simulink model "BMSModel"  (R2024b)
settings: Solver=VariableStepAuto  StartTime=0.0  StopTime=+inf  RelTol=1e-3
values from neighbouring files (symbols this model uses):
  CellCurrentLimitThreshold_Fault = 50    [startInit.m]
  CellCurrentLimitThreshold_Warning = 20    [startInit.m]
  CellTemperatureLimitThreshold_Fault = 65    [startInit.m]
  CellTemperatureLimitThreshold_Warning = 50    [startInit.m]
  CellVoltageLimitHigh_Fault = 4.25    [startInit.m]
  CellVoltageLimitHigh_Warning = 4.21    [startInit.m]
  CellVoltageLimitLow_Fault = 2.65    [startInit.m]
  CellVoltageLimitLow_Warning = 2.70    (= 2.7)    [startInit.m]
  ChargerParRes = 10000    [startInit.m]
  ChargerRes = 0    [startInit.m]
  DisChargerRes = 250    [startInit.m]
  DisInverterRes = 250    [startInit.m]
  DrivetrainEnDelay = 0.1    [startInit.m]
  InverterParRes = 10000    [startInit.m]
  InverterRes = 0    [startInit.m]
  PreChargerRes = 1000    [startInit.m]
  PreInverterRes = 1000    [startInit.m]
  VbattMin = 1    [startInit.m]
  VbattThersholdChrg = 0.8    [startInit.m]
  VbattThresholdDis = 0.2    [startInit.m]
undefined symbols (not defined in the file or in its folder): ContactorCmd, DisChrgCmd, DrivetrainEn, PassiveBalancingRes, PreChrgCmd, sigbuilder_block
libraries: fl_lib x135, nesl_utility x91, deeplib x11, batteryecm_lib x10, ee_lib x10
(part 2 of 13)

== system BMSModel/Electric Vehicul Model/HV Battery/Battery Pack/Battery Model ==
  AmbTemp : Inport
  ShortFaultCmd : Inport  Port=2
  OpenFaultCmd : Inport  Port=3
  PassiveControlBalCmd : Inport  Port=4
  Battery_Model : Subsystem
  Resistor : fl_lib/Electrical/Electrical Elements/Resistor  R=0.05 Ohm  i_specify=off  i_priority=None  i=0 A  i_nominal_specify=off  i_nominal_value=1  i_nominal_unit=A  v_specify=off  v_priority=None  v=0 V  v_nominal_specify=off  v_nominal_value=1  v_nominal_unit=V
  Resistor1 : fl_lib/Electrical/Electrical Elements/Resistor  R=0.05 Ohm  i_specify=off  i_priority=None  i=0 A  i_nominal_specify=off  i_nominal_value=1  i_nominal_unit=A  v_specify=off  v_priority=None  v=0 V  v_nominal_specify=off  v_nominal_value=1  v_nominal_unit=V
  Subsystem1 : Subsystem
  BattMod+ : PMIOPort  Side=Right
  BattMod- : PMIOPort  Port=2  Side=Right
  TCellVector : Outport
  RealSOCVector : Outport  Port=2
  VoltageVector : Outport  Port=3
  CurrentCellVector : Outport  Port=4
  wires:
  OpenFaultCmd/1 -> Battery_Model/3(OpenFaultCmd)
  Battery_Model/1(TCellVector) -> TCellVector/1
  Subsystem1/1(VoltageVector) -> VoltageVector/1
  Battery_Model/2(RealSocVector) -> RealSOCVector/1
  Battery_Model/3(CurrentCellVector) -> CurrentCellVector/1
  AmbTemp/1 -> Battery_Model/1(AmbTemp)
  PassiveControlBalCmd/1 -> Subsystem1/1(PassiveControlBalCmd1)
  ShortFaultCmd/1 -> Battery_Model/2(ShortFaultCmd)
  connections:
  Subsystem1/LConn7 -- Battery_Model/RConn7
  Subsystem1/LConn1 -- Resistor/LConn1 -- Battery_Model/RConn1
  Subsystem1/LConn11 -- Resistor1/LConn1 -- Battery_Model/RConn11
  Subsystem1/LConn9 -- Battery_Model/RConn9
  Subsystem1/LConn6 -- Battery_Model/RConn6
  Subsystem1/LConn10 -- Battery_Model/RConn10
  Subsystem1/LConn4 -- Battery_Model/RConn4
  Subsystem1/LConn5 -- Battery_Model/RConn5
  Subsystem1/LConn8 -- Battery_Model/RConn8
  Subsystem1/LConn3 -- Battery_Model/RConn3
  Subsystem1/LConn2 -- Battery_Model/RConn2
  BattMod+/RConn1 -- Resistor/RConn1
  BattMod-/RConn1 -- Resistor1/RConn1

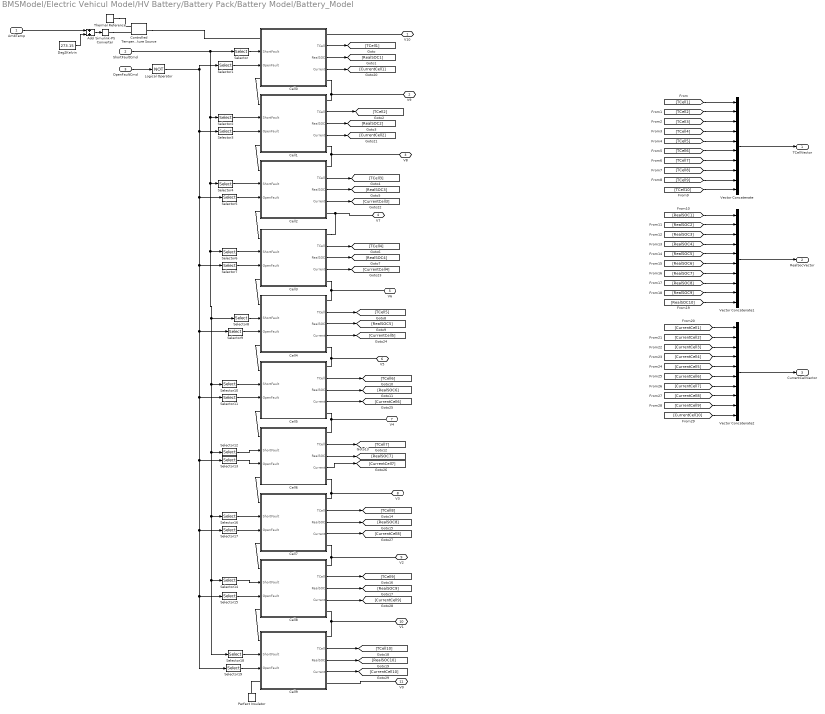
== system BMSModel/Electric Vehicul Model/HV Battery/Battery Pack/Battery Model/Battery_Model ==
  AmbTemp : Inport
  ShortFaultCmd : Inport  Port=2
  OpenFaultCmd : Inport  Port=3  OutDataTypeStr=boolean
  Add : Sum
  Cell0 : Subsystem
      settings: PortSchema="{\"entries\":[{\"content\":{\"sides\":[{\"content\":{\"connectorIds\":[\"LConn1\",\"In1\",\"In2\",\"LConn2\"]},\"type\":\"ConnectorPlacement.EquallySpacedRectSide\",\"uuid\":\"beb41537-88d3-447c-be57-cb35454cee64\"},{\"content\":{\"connectorIds\":[\"RConn1\",\"Out1\",\"Out2\",\"Out3\",\"RConn2\"],\"side\":\"RIGHT\"},\"type\":\"ConnectorPlacement.EquallySpacedRectSide\",\"uuid\":\"6a20e019-ad86-49c6-8fd8-d46a955d3cd5\"},{\"content\":{\"side\":\"TOP\"},\"type\":\"ConnectorPlacement.EquallySpacedRectSide\",\"uuid\":\"023fac5e-8c8d-4c82-a029-53fce6daf92f\"}]},\"type\":\"ConnectorPlacement.EquallySpacedConnector\",\"uuid\":\"8f1c4e8b-ec41-4c09-b75c-bd6301f7044f\"}],\"packageUris\":[\"http://schema.mathworks.com/mf0/Connector/R2020b\"],\"version\":\"1.0\"}"
      mask Display: image('.\icons\images.jpg');
  Cell1 : Subsystem
      settings: PortSchema="{\"entries\":[{\"content\":{\"sides\":[{\"content\":{\"connectorIds\":[\"LConn1\",\"In1\",\"In2\",\"LConn2\"]},\"type\":\"ConnectorPlacement.EquallySpacedRectSide\",\"uuid\":\"beb41537-88d3-447c-be57-cb35454cee64\"},{\"content\":{\"connectorIds\":[\"RConn1\",\"Out1\",\"Out2\",\"Out3\",\"RConn2\"],\"side\":\"RIGHT\"},\"type\":\"ConnectorPlacement.EquallySpacedRectSide\",\"uuid\":\"6a20e019-ad86-49c6-8fd8-d46a955d3cd5\"},{\"content\":{\"side\":\"TOP\"},\"type\":\"ConnectorPlacement.EquallySpacedRectSide\",\"uuid\":\"023fac5e-8c8d-4c82-a029-53fce6daf92f\"}]},\"type\":\"ConnectorPlacement.EquallySpacedConnector\",\"uuid\":\"8f1c4e8b-ec41-4c09-b75c-bd6301f7044f\"}],\"packageUris\":[\"http://schema.mathworks.com/mf0/Connector/R2020b\"],\"version\":\"1.0\"}"
      mask Display: image('.\icons\images.jpg');
  Cell2 : Subsystem
      settings: PortSchema="{\"entries\":[{\"content\":{\"sides\":[{\"content\":{\"connectorIds\":[\"LConn1\",\"In1\",\"In2\",\"LConn2\"]},\"type\":\"ConnectorPlacement.EquallySpacedRectSide\",\"uuid\":\"beb41537-88d3-447c-be57-cb35454cee64\"},{\"content\":{\"connectorIds\":[\"RConn1\",\"Out1\",\"Out2\",\"Out3\",\"RConn2\"],\"side\":\"RIGHT\"},\"type\":\"ConnectorPlacement.EquallySpacedRectSide\",\"uuid\":\"6a20e019-ad86-49c6-8fd8-d46a955d3cd5\"},{\"content\":{\"side\":\"TOP\"},\"type\":\"ConnectorPlacement.EquallySpacedRectSide\",\"uuid\":\"023fac5e-8c8d-4c82-a029-53fce6daf92f\"}]},\"type\":\"ConnectorPlacement.EquallySpacedConnector\",\"uuid\":\"8f1c4e8b-ec41-4c09-b75c-bd6301f7044f\"}],\"packageUris\":[\"http://schema.mathworks.com/mf0/Connector/R2020b\"],\"version\":\"1.0\"}"
      mask Display: image('D:\BMS-AI\Dev_BMS_with_AI\icons\images.jpg');
  Cell3 : Subsystem
      settings: PortSchema="{\"entries\":[{\"content\":{\"sides\":[{\"content\":{\"connectorIds\":[\"LConn1\",\"In1\",\"In2\",\"LConn2\"]},\"type\":\"ConnectorPlacement.EquallySpacedRectSide\",\"uuid\":\"beb41537-88d3-447c-be57-cb35454cee64\"},{\"content\":{\"connectorIds\":[\"RConn1\",\"Out1\",\"Out2\",\"Out3\",\"RConn2\"],\"side\":\"RIGHT\"},\"type\":\"ConnectorPlacement.EquallySpacedRectSide\",\"uuid\":\"6a20e019-ad86-49c6-8fd8-d46a955d3cd5\"},{\"content\":{\"side\":\"TOP\"},\"type\":\"ConnectorPlacement.EquallySpacedRectSide\",\"uuid\":\"023fac5e-8c8d-4c82-a029-53fce6daf92f\"}]},\"type\":\"ConnectorPlacement.EquallySpacedConnector\",\"uuid\":\"8f1c4e8b-ec41-4c09-b75c-bd6301f7044f\"}],\"packageUris\":[\"http://schema.mathworks.com/mf0/Connector/R2020b\"],\"version\":\"1.0\"}"
  Cell4 : Subsystem
      settings: PortSchema="{\"entries\":[{\"content\":{\"sides\":[{\"content\":{\"connectorIds\":[\"LConn1\",\"In1\",\"In2\",\"LConn2\"]},\"type\":\"ConnectorPlacement.EquallySpacedRectSide\",\"uuid\":\"beb41537-88d3-447c-be57-cb35454cee64\"},{\"content\":{\"connectorIds\":[\"RConn1\",\"Out1\",\"Out2\",\"Out3\",\"RConn2\"],\"side\":\"RIGHT\"},\"type\":\"ConnectorPlacement.EquallySpacedRectSide\",\"uuid\":\"6a20e019-ad86-49c6-8fd8-d46a955d3cd5\"},{\"content\":{\"side\":\"TOP\"},\"type\":\"ConnectorPlacement.EquallySpacedRectSide\",\"uuid\":\"023fac5e-8c8d-4c82-a029-53fce6daf92f\"}]},\"type\":\"ConnectorPlacement.EquallySpacedConnector\",\"uuid\":\"8f1c4e8b-ec41-4c09-b75c-bd6301f7044f\"}],\"packageUris\":[\"http://schema.mathworks.com/mf0/Connector/R2020b\"],\"version\":\"1.0\"}"
  Cell5 : Subsystem
      settings: PortSchema="{\"entries\":[{\"content\":{\"sides\":[{\"content\":{\"connectorIds\":[\"LConn1\",\"In1\",\"In2\",\"LConn2\"]},\"type\":\"ConnectorPlacement.EquallySpacedRectSide\",\"uuid\":\"beb41537-88d3-447c-be57-cb35454cee64\"},{\"content\":{\"connectorIds\":[\"RConn1\",\"Out1\",\"Out2\",\"Out3\",\"RConn2\"],\"side\":\"RIGHT\"},\"type\":\"ConnectorPlacement.EquallySpacedRectSide\",\"uuid\":\"6a20e019-ad86-49c6-8fd8-d46a955d3cd5\"},{\"content\":{\"side\":\"TOP\"},\"type\":\"ConnectorPlacement.EquallySpacedRectSide\",\"uuid\":\"023fac5e-8c8d-4c82-a029-53fce6daf92f\"}]},\"type\":\"ConnectorPlacement.EquallySpacedConnector\",\"uuid\":\"8f1c4e8b-ec41-4c09-b75c-bd6301f7044f\"}],\"packageUris\":[\"http://schema.mathworks.com/mf0/Connector/R2020b\"],\"version\":\"1.0\"}"
  Cell6 : Subsystem
      settings: PortSchema="{\"entries\":[{\"content\":{\"sides\":[{\"content\":{\"connectorIds\":[\"LConn1\",\"In1\",\"In2\",\"LConn2\"]},\"type\":\"ConnectorPlacement.EquallySpacedRectSide\",\"uuid\":\"beb41537-88d3-447c-be57-cb35454cee64\"},{\"content\":{\"connectorIds\":[\"RConn1\",\"Out1\",\"Out2\",\"Out3\",\"RConn2\"],\"side\":\"RIGHT\"},\"type\":\"ConnectorPlacement.EquallySpacedRectSide\",\"uuid\":\"6a20e019-ad86-49c6-8fd8-d46a955d3cd5\"},{\"content\":{\"side\":\"TOP\"},\"type\":\"ConnectorPlacement.EquallySpacedRectSide\",\"uuid\":\"023fac5e-8c8d-4c82-a029-53fce6daf92f\"}]},\"type\":\"ConnectorPlacement.EquallySpacedConnector\",\"uuid\":\"8f1c4e8b-ec41-4c09-b75c-bd6301f7044f\"}],\"packageUris\":[\"http://schema.mathworks.com/mf0/Connector/R2020b\"],\"version\":\"1.0\"}"
  Cell7 : Subsystem
      settings: PortSchema="{\"entries\":[{\"content\":{\"sides\":[{\"content\":{\"connectorIds\":[\"LConn1\",\"In1\",\"In2\",\"LConn2\"]},\"type\":\"ConnectorPlacement.EquallySpacedRectSide\",\"uuid\":\"beb41537-88d3-447c-be57-cb35454cee64\"},{\"content\":{\"connectorIds\":[\"RConn1\",\"Out1\",\"Out2\",\"Out3\",\"RConn2\"],\"side\":\"RIGHT\"},\"type\":\"ConnectorPlacement.EquallySpacedRectSide\",\"uuid\":\"6a20e019-ad86-49c6-8fd8-d46a955d3cd5\"},{\"content\":{\"side\":\"TOP\"},\"type\":\"ConnectorPlacement.EquallySpacedRectSide\",\"uuid\":\"023fac5e-8c8d-4c82-a029-53fce6daf92f\"}]},\"type\":\"ConnectorPlacement.EquallySpacedConnector\",\"uuid\":\"8f1c4e8b-ec41-4c09-b75c-bd6301f7044f\"}],\"packageUris\":[\"http://schema.mathworks.com/mf0/Connector/R2020b\"],\"version\":\"1.0\"}"
  Cell8 : Subsystem
      settings: PortSchema="{\"entries\":[{\"content\":{\"sides\":[{\"content\":{\"connectorIds\":[\"LConn1\",\"In1\",\"In2\",\"LConn2\"]},\"type\":\"ConnectorPlacement.EquallySpacedRectSide\",\"uuid\":\"beb41537-88d3-447c-be57-cb35454cee64\"},{\"content\":{\"connectorIds\":[\"RConn1\",\"Out1\",\"Out2\",\"Out3\",\"RConn2\"],\"side\":\"RIGHT\"},\"type\":\"ConnectorPlacement.EquallySpacedRectSide\",\"uuid\":\"6a20e019-ad86-49c6-8fd8-d46a955d3cd5\"},{\"content\":{\"side\":\"TOP\"},\"type\":\"ConnectorPlacement.EquallySpacedRectSide\",\"uuid\":\"023fac5e-8c8d-4c82-a029-53fce6daf92f\"}]},\"type\":\"ConnectorPlacement.EquallySpacedConnector\",\"uuid\":\"8f1c4e8b-ec41-4c09-b75c-bd6301f7044f\"}],\"packageUris\":[\"http://schema.mathworks.com/mf0/Connector/R2020b\"],\"version\":\"1.0\"}"
  Cell9 : Subsystem
      settings: PortSchema="{\"entries\":[{\"content\":{\"sides\":[{\"content\":{\"connectorIds\":[\"LConn1\",\"In1\",\"In2\",\"LConn2\"]},\"type\":\"ConnectorPlacement.EquallySpacedRectSide\",\"uuid\":\"beb41537-88d3-447c-be57-cb35454cee64\"},{\"content\":{\"connectorIds\":[\"RConn1\",\"Out1\",\"Out2\",\"Out3\",\"RConn2\"],\"side\":\"RIGHT\"},\"type\":\"ConnectorPlacement.EquallySpacedRectSide\",\"uuid\":\"6a20e019-ad86-49c6-8fd8-d46a955d3cd5\"},{\"content\":{\"side\":\"TOP\"},\"type\":\"ConnectorPlacement.EquallySpacedRectSide\",\"uuid\":\"023fac5e-8c8d-4c82-a029-53fce6daf92f\"}]},\"type\":\"ConnectorPlacement.EquallySpacedConnector\",\"uuid\":\"8f1c4e8b-ec41-4c09-b75c-bd6301f7044f\"}],\"packageUris\":[\"http://schema.mathworks.com/mf0/Connector/R2020b\"],\"version\":\"1.0\"}"
  Controlled Temperature Source : fl_lib/Thermal/Thermal Sources/Controlled Temperature Source
  Deg2Kelvin : Constant  Value=273.15
  Logical Operator : Logic  Operator=NOT  AllPortsSameDT=off  OutDataTypeStr=boolean
  Perfect Insulator : fl_lib/Thermal/Thermal Elements/Perfect Insulator  T_specify=off  T_priority=None  T=293.15 K  T_nominal_specify=off  T_nominal_value=1  T_nominal_unit=K
  Selector : Selector  InputPortWidth=10  IndexOptions=Index vector (dialog)  Indices=1  OutputSizes=1
  Selector1 : Selector  InputPortWidth=10  IndexOptions=Index vector (dialog)  Indices=1  OutputSizes=1
  Selector10 : Selector  InputPortWidth=10  IndexOptions=Index vector (dialog)  Indices=6  OutputSizes=1
  Selector11 : Selector  InputPortWidth=10  IndexOptions=Index vector (dialog)  Indices=6  OutputSizes=1
  Selector12 : Selector  InputPortWidth=10  IndexOptions=Index vector (dialog)  Indices=7  OutputSizes=1
  Selector13 : Selector  InputPortWidth=10  IndexOptions=Index vector (dialog)  Indices=7  OutputSizes=1
  Selector14 : Selector  InputPortWidth=10  IndexOptions=Index vector (dialog)  Indices=9  OutputSizes=1
  Selector15 : Selector  InputPortWidth=10  IndexOptions=Index vector (dialog)  Indices=9  OutputSizes=1
  Selector16 : Selector  InputPortWidth=10  IndexOptions=Index vector (dialog)  Indices=8  OutputSizes=1
  Selector17 : Selector  InputPortWidth=10  IndexOptions=Index vector (dialog)  Indices=8  OutputSizes=1
  Selector18 : Selector  InputPortWidth=10  IndexOptions=Index vector (dialog)  Indices=10  OutputSizes=1
  Selector19 : Selector  InputPortWidth=10  IndexOptions=Index vector (dialog)  Indices=10  OutputSizes=1
  Selector2 : Selector  InputPortWidth=10  IndexOptions=Index vector (dialog)  Indices=2  OutputSizes=1
  Selector3 : Selector  InputPortWidth=10  IndexOptions=Index vector (dialog)  Indices=2  OutputSizes=1
  Selector4 : Selector  InputPortWidth=10  IndexOptions=Index vector (dialog)  Indices=3  OutputSizes=1
  Selector5 : Selector  InputPortWidth=10  IndexOptions=Index vector (dialog)  Indices=3  OutputSizes=1
  Selector6 : Selector  InputPortWidth=10  IndexOptions=Index vector (dialog)  Indices=4  OutputSizes=1
  Selector7 : Selector  InputPortWidth=10  IndexOptions=Index vector (dialog)  Indices=4  OutputSizes=1
  Selector8 : Selector  InputPortWidth=10  IndexOptions=Index vector (dialog)  Indices=5  OutputSizes=1
  Selector9 : Selector  InputPortWidth=10  IndexOptions=Index vector (dialog)  Indices=5  OutputSizes=1
  Simulink-PS Converter : nesl_utility/Simulink-PS Converter  PhysicalDomain=network_engine_domain  SubClassName=ps_input  LeftPortType=input  RightPortType=output  PseudoPeriodic=off  Frequencies=[]  NoiseDistribution=none  NoiseParameters=[]  Unit=1  AffineConversion=off  FilteringAndDerivatives=provide  SimscapeFilterOrder=1  InputFilterTimeConstant=0.001  UdotUserProvided=0
  Thermal Reference : fl_lib/Thermal/Thermal Elements/Thermal Reference
  Vector Concatenate : Concatenate  NumInputs=10
  Vector Concatenate1 : Concatenate  NumInputs=10
  Vector Concatenate2 : Concatenate  NumInputs=10
  V10 : PMIOPort  Side=Right
  V9 : PMIOPort  Port=2  Side=Right
  V8 : PMIOPort  Port=3  Side=Right
  V7 : PMIOPort  Port=4  Side=Right
  V6 : PMIOPort  Port=5  Side=Right
  V5 : PMIOPort  Port=6  Side=Right
  V4 : PMIOPort  Port=7  Side=Right
  V3 : PMIOPort  Port=8  Side=Right
  V2 : PMIOPort  Port=9  Side=Right
  V1 : PMIOPort  Port=10  Side=Right
  V0 : PMIOPort  Port=11  Side=Right
  TCellVector : Outport
  RealSocVector : Outport  Port=2
  CurrentCellVector : Outport  Port=3
  wires:
  Deg2Kelvin/1 -> Add/2
  Add/1 -> Simulink-PS Converter/1
  AmbTemp/1 -> Add/1
  Cell0/1(TCell) -> [TCell1]
  Cell0/2(RealSOC) -> [RealSOC1]
  Cell0/3(Current) -> [CurrentCell1]
  Cell1/1(TCell) -> [TCell2]
  Cell1/2(RealSOC) -> [RealSOC2]
  Cell1/3(Current) -> [CurrentCell2]
  Cell2/1(TCell) -> [TCell3]
  Cell2/2(RealSOC) -> [RealSOC3]
  Cell2/3(Current) -> [CurrentCell3]
  Cell3/1(TCell) -> [TCell4]
  Cell3/2(RealSOC) -> [RealSOC4]
  Cell3/3(Current) -> [CurrentCell4]
  Cell4/1(TCell) -> [TCell5]
  Cell4/2(RealSOC) -> [RealSOC5]
  Cell4/3(Current) -> [CurrentCell5]
  Cell5/1(TCell) -> [TCell6]
  Cell5/2(RealSOC) -> [RealSOC6]
  Cell5/3(Current) -> [CurrentCell6]
  Cell6/1(TCell) -> [TCell7]
  Cell6/2(RealSOC) -> [RealSOC7]
  Cell6/3(Current) -> [CurrentCell7]
  Cell7/2(RealSOC) -> [RealSOC8]
  Cell7/1(TCell) -> [TCell8]
  Cell7/3(Current) -> [CurrentCell8]
  Cell8/2(RealSOC) -> [RealSOC9]
  Cell8/1(TCell) -> [TCell9]
  Cell8/3(Current) -> [CurrentCell9]
  Cell9/2(RealSOC) -> [RealSOC10]
  Cell9/1(TCell) -> [TCell10]
  Cell9/3(Current) -> [CurrentCell10]
  OpenFaultCmd/1 -> Logical Operator/1
  Logical Operator/1 -> Selector3/1, Selector5/1, Selector7/1, Selector19/1, Selector15/1, Selector17/1, Selector13/1, Selector11/1, Selector9/1, Selector1/1
  ShortFaultCmd/1 -> Selector6/1, Selector8/1, Selector10/1, Selector18/1, Selector14/1, Selector16/1, Selector12/1, Selector4/1, Selector2/1, Selector/1
  Selector/1 -> Cell0/1(ShortFault)
  Selector1/1 -> Cell0/2(OpenFault)
  Selector2/1 -> Cell1/1(ShortFault)
  Selector3/1 -> Cell1/2(OpenFault)
  Selector4/1 -> Cell2/1(ShortFault)
  Selector5/1 -> Cell2/2(OpenFault)
  Selector6/1 -> Cell3/1(ShortFault)
  Selector7/1 -> Cell3/2(OpenFault)
  Selector8/1 -> Cell4/1(ShortFault)
  Selector9/1 -> Cell4/2(OpenFault)
  Selector10/1 -> Cell5/1(ShortFault)
  Selector11/1 -> Cell5/2(OpenFault)
  Selector12/1 -> Cell6/1(ShortFault)
  Selector13/1 -> Cell6/2(OpenFault)
  Selector16/1 -> Cell7/1(ShortFault)
  Selector17/1 -> Cell7/2(OpenFault)
  Selector14/1 -> Cell8/1(ShortFault)
  Selector15/1 -> Cell8/2(OpenFault)
  Selector18/1 -> Cell9/1(ShortFault)
  Selector19/1 -> Cell9/2(OpenFault)
  Vector Concatenate/1 -> TCellVector/1
  [TCell1] -> Vector Concatenate/1
  [TCell2] -> Vector Concatenate/2
  [TCell3] -> Vector Concatenate/3
  [TCell4] -> Vector Concatenate/4
  [TCell5] -> Vector Concatenate/5
  [TCell6] -> Vector Concatenate/6
  [TCell7] -> Vector Concatenate/7
  [TCell8] -> Vector Concatenate/8
  [TCell9] -> Vector Concatenate/9
  [TCell10] -> Vector Concatenate/10
  Vector Concatenate1/1 -> RealSocVector/1
  [RealSOC1] -> Vector Concatenate1/1
  [RealSOC2] -> Vector Concatenate1/2
  [RealSOC3] -> Vector Concatenate1/3
  [RealSOC4] -> Vector Concatenate1/4
  [RealSOC5] -> Vector Concatenate1/5
  [RealSOC6] -> Vector Concatenate1/6
  [RealSOC7] -> Vector Concatenate1/7
  [RealSOC8] -> Vector Concatenate1/8
  [RealSOC9] -> Vector Concatenate1/9
  [RealSOC10] -> Vector Concatenate1/10
  Vector Concatenate2/1 -> CurrentCellVector/1
  [CurrentCell1] -> Vector Concatenate2/1
  [CurrentCell2] -> Vector Concatenate2/2
  [CurrentCell3] -> Vector Concatenate2/3
  [CurrentCell4] -> Vector Concatenate2/4
  [CurrentCell5] -> Vector Concatenate2/5
  [CurrentCell6] -> Vector Concatenate2/6
  [CurrentCell7] -> Vector Concatenate2/7
  [CurrentCell8] -> Vector Concatenate2/8
  [CurrentCell9] -> Vector Concatenate2/9
  [CurrentCell10] -> Vector Concatenate2/10
  connections:
  Simulink-PS Converter/RConn1 -- Controlled Temperature Source/RConn1
  Thermal Reference/LConn1 -- Controlled Temperature Source/RConn2
  Cell0/LConn2 -- Cell1/LConn1
  Cell0/RConn2 -- V9/RConn1 -- Cell1/RConn1
  Cell1/LConn2 -- Cell2/LConn1
  Cell1/RConn2 -- Cell2/RConn1 -- V8/RConn1
  Cell4/LConn2 -- Cell5/LConn1
  Cell3/RConn2 -- V6/RConn1 -- Cell4/RConn1
  Cell4/RConn2 -- V5/RConn1 -- Cell5/RConn1
  Cell3/LConn2 -- Cell4/LConn1
  Cell6/LConn2 -- Cell7/LConn1
  Cell6/RConn2 -- V3/RConn1 -- Cell7/RConn1
  Cell7/RConn2 -- V2/RConn1 -- Cell8/RConn1
  Cell7/LConn2 -- Cell8/LConn1
  V10/RConn1 -- Cell0/RConn1
  V0/RConn1 -- Cell9/RConn2
  V7/RConn1 -- Cell3/RConn1 -- Cell2/RConn2
  Cell2/LConn2 -- Cell3/LConn1
  Cell5/RConn2 -- Cell6/RConn1 -- V4/RConn1
  Cell5/LConn2 -- Cell6/LConn1
  Cell9/RConn1 -- V1/RConn1 -- Cell8/RConn2
  Cell8/LConn2 -- Cell9/LConn1
  Controlled Temperature Source/LConn1 -- Cell0/LConn1
  Perfect Insulator/LConn1 -- Cell9/LConn2

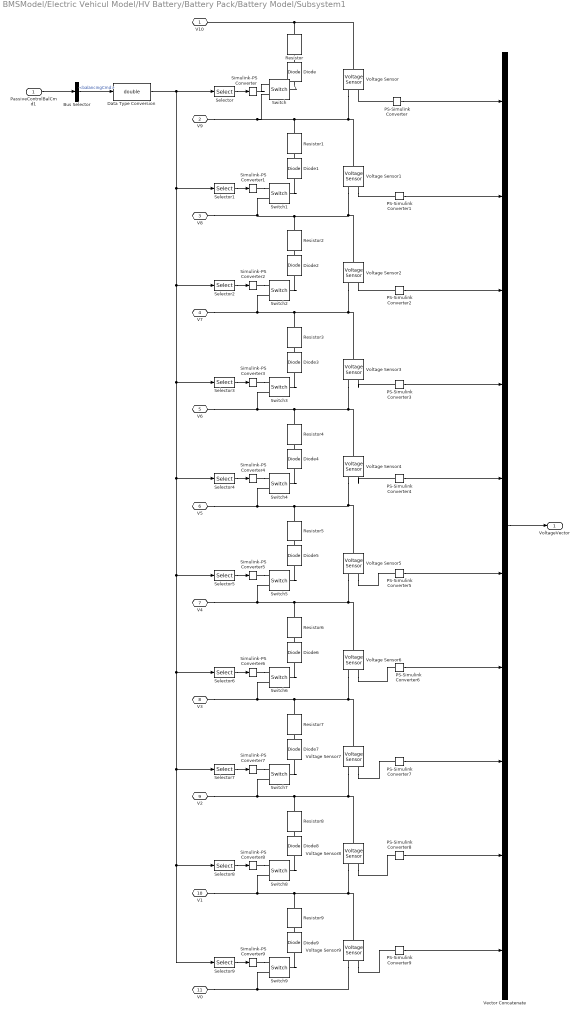
== system BMSModel/Electric Vehicul Model/HV Battery/Battery Pack/Battery Model/Subsystem1 ==
  PassiveControlBalCmd1 : Inport  OutDataTypeStr=boolean
  Bus Selector : BusSelector  OutputSignals=Balancing.balancingCmd
  Data Type Conversion : DataTypeConversion  OutDataTypeStr=double  SaturateOnIntegerOverflow=off  RndMeth=Floor
  Diode{,1..9} (10 alike) : ee_lib/Semiconductors & Converters/Diode  ModelType=ee.enum.diode.modelType.pwl  TableType=ee.enum.diode.tableType.IfMat  IfMat=[.07, .12, .19, 1.75, 4.24, 7.32, 11.2; .16, .3, .72, 2.14, 4.02, 6.35, 9.12] A  VfMat=[.9, 1.15, 1.25, 1.5, 1.75, 2.17, 2.6, 2.85; .58, .68, .75, 1.1, 1.38, 1.77, 2.27, 2.7] V  TjVec=[25, 125] degC  VfVec=[.5, .7, .9, 1.3, 1.7, 2.1, 2.5] V  IfVec=[.1, .2, .5, 1, 2, 4, 7, 10] A  IrMat=[1e-06, 2e-06, 5e-06, 1e-05, 2.5e-05, 5e-05, .0001; 2e-06, 5e-06, 1e-05, 4e-05, 8e-05, .0002, .0005] A  VrMat=[10, 100, 200, 250, 300, 325, 350; 10, 70, 160, 220, 270, 300, 320] V  VrVec=[10, 20, 40, 50, 70, 80, 100] V  IrVec=[1e-06, 2e-06, 5e-06, 1e-05, 2e-05, 5e-05, .0001] A  Vf=0.6 V  Ron=0.3 Ohm  Goff=1e-8 S  prmExp=ee.enum.diode.expParam.useTwo  I12=[.0137, .545] A  V12=[.6, .7] V  RS=0 Ohm  Tmeas=25 degC  IS=1e-12 A  ec=1  I1=0.0137 A  V1=0.6 V  N_series=1  N_parallel=1  ZenerEnable=false  Rz=0.3 Ohm  BV=inf V  C_param=ee.enum.diode.capParam.fixed  CJ=5 pF  CJ0=5 pF  Vvec=[.1, 10, 100] V  Cvec=[3.5, 1, .4] pF  VJ=1 V  Mgrad=0.5  FC=0.5  Q_param=ee.enum.diode.recoveryParam.off  iRM=-7.15 A  iF=4 A  didt=-750 A/us  trr_factor=3  trr=115 ns  Qrr=150 s*nA  Vr=-600 V  Ls=150 nH  Erec=0.03 J  TT=50 ns  tau=100 ns  T_param=ee.enum.diode.temperatureParam.off  Tdevice=25 degC  I1_T2=0.245 A  V1_T2=0.5 V  IS_T2=1.25e-7 A  Tmeas2=125 degC  XTI_param=ee.enum.diode.xtiParam.pn  XTI=3  EG_param=ee.enum.diode.egapParam.material_si  EG=1.11 eV  dBVdT=0 V/K  fault_action=simscape.enum.assert.action.none  t_fault=inf s  enable_fault_input=simscape.enum.onoff.off  i_specify=off  i_priority=None  i=0 A  i_nominal_specify=off  i_nominal_value=1  i_nominal_unit=A  v_specify=off  v_priority=None  v=0 V  v_nominal_specify=off  v_nominal_value=1  v_nominal_unit=V  v_capacitor_specify=off  v_capacitor_priority=None  v_capacitor=0 V  v_capacitor_nominal_specify=off  v_capacitor_nominal_value=1  v_capacitor_nominal_unit=V  Qj_specify=off  Qj_priority=High  Qj=0 C  Qj_nominal_specify=off  Qj_nominal_value=1  Qj_nominal_unit=C  i_diode_specify=off  i_diode_priority=None  i_diode=0 A  i_diode_nominal_specify=off  i_diode_nominal_value=1  i_diode_nominal_unit=A  v_diode_specify=off  v_diode_priority=None  v_diode=0 V  v_diode_nominal_specify=off  v_diode_nominal_value=1  v_diode_nominal_unit=V  i_capacitor_specify=off  i_capacitor_priority=None  i_capacitor=0 A  i_capacitor_nominal_specify=off  i_capacitor_nominal_value=1  i_capacitor_nominal_unit=A  power_dissipated_specify=off  power_dissipated_priority=None  power_dissipated=0 W  power_dissipated_nominal_specify=off  power_dissipated_nominal_value=1  power_dissipated_nominal_unit=W  fidelity_level=ee.enum.diode.fidelity.includeChargeDyn  rec_loss_model=ee.enum.diode.reverseRecoveryModel.fixed  ErecVal=0 J  scaleWithIandV=true  ErecMat=zeros(2, 3) J  TjrecVec=[298.15, 398.15] K  IfrecVec=[.1, 1, 10] A  IrecVal=10 A  VrecVal=10 V  tFilter=1 / 1000 / 100 s  thermal_network_parameterization=ee.enum.thermalNetworkTopology.cauer  Rth=[0, 10] K/W  Rth_Cauer=[1, 3, 10] K/W  Rth_Foster=[4, 6] K/W  thermal_mass_parameterization=ee.enum.thermalNetwork.timeconstant  thermal_mass=[0, 1] J/K  thermal_mass_Cauer=[.1, .3, 1] J/K  thermal_mass_Foster=[1.5, 3] J/K  thermal_time_constant=[0, 10] s  thermal_time_constant_Cauer=[1, 3, 10] s  thermal_time_constant_Foster=[6, 18] s  thermal_mass_initial_temperatures=[25, 25] degC  thermal_mass_initial_temperatures_Cauer=[25, 25, 25] degC  junction_thermal_mass=0.01 J/K  Tvec_initial_Foster=[25, 25, 25, 25] degC  no_thermal_network=0  junction_temperature_specify=off  junction_temperature_priority=None  junction_temperature=25 degC  junction_temperature_nominal_specify=off  junction_temperature_nominal_value=1  junction_temperature_nominal_unit=degC
  PS-Simulink Converter{,1..9} (10 alike) : nesl_utility/PS-Simulink Converter  PhysicalDomain=network_engine_domain  SubClassName=ps_output  LeftPortType=input  RightPortType=output  PseudoPeriodic=off  Frequencies=[]  VectorFormat=1-D array  Unit=inherit  AffineConversion=off
  Resistor : fl_lib/Electrical/Electrical Elements/Resistor  R=PassiveBalancingRes(1) Ohm  i_specify=off  i_priority=None  i=0 A  i_nominal_specify=off  i_nominal_value=1  i_nominal_unit=A  v_specify=off  v_priority=None  v=0 V  v_nominal_specify=off  v_nominal_value=1  v_nominal_unit=V
  Resistor1 : fl_lib/Electrical/Electrical Elements/Resistor  R=PassiveBalancingRes(2) Ohm  i_specify=off  i_priority=None  i=0 A  i_nominal_specify=off  i_nominal_value=1  i_nominal_unit=A  v_specify=off  v_priority=None  v=0 V  v_nominal_specify=off  v_nominal_value=1  v_nominal_unit=V
  Resistor2 : fl_lib/Electrical/Electrical Elements/Resistor  R=PassiveBalancingRes(3) Ohm  i_specify=off  i_priority=None  i=0 A  i_nominal_specify=off  i_nominal_value=1  i_nominal_unit=A  v_specify=off  v_priority=None  v=0 V  v_nominal_specify=off  v_nominal_value=1  v_nominal_unit=V
  Resistor3 : fl_lib/Electrical/Electrical Elements/Resistor  R=PassiveBalancingRes(4) Ohm  i_specify=off  i_priority=None  i=0 A  i_nominal_specify=off  i_nominal_value=1  i_nominal_unit=A  v_specify=off  v_priority=None  v=0 V  v_nominal_specify=off  v_nominal_value=1  v_nominal_unit=V
  Resistor4 : fl_lib/Electrical/Electrical Elements/Resistor  R=PassiveBalancingRes(5) Ohm  i_specify=off  i_priority=None  i=0 A  i_nominal_specify=off  i_nominal_value=1  i_nominal_unit=A  v_specify=off  v_priority=None  v=0 V  v_nominal_specify=off  v_nominal_value=1  v_nominal_unit=V
  Resistor5 : fl_lib/Electrical/Electrical Elements/Resistor  R=PassiveBalancingRes(6) Ohm  i_specify=off  i_priority=None  i=0 A  i_nominal_specify=off  i_nominal_value=1  i_nominal_unit=A  v_specify=off  v_priority=None  v=0 V  v_nominal_specify=off  v_nominal_value=1  v_nominal_unit=V
  Resistor6 : fl_lib/Electrical/Electrical Elements/Resistor  R=PassiveBalancingRes(7) Ohm  i_specify=off  i_priority=None  i=0 A  i_nominal_specify=off  i_nominal_value=1  i_nominal_unit=A  v_specify=off  v_priority=None  v=0 V  v_nominal_specify=off  v_nominal_value=1  v_nominal_unit=V
  Resistor7 : fl_lib/Electrical/Electrical Elements/Resistor  R=PassiveBalancingRes(8) Ohm  i_specify=off  i_priority=None  i=0 A  i_nominal_specify=off  i_nominal_value=1  i_nominal_unit=A  v_specify=off  v_priority=None  v=0 V  v_nominal_specify=off  v_nominal_value=1  v_nominal_unit=V
  Resistor8 : fl_lib/Electrical/Electrical Elements/Resistor  R=PassiveBalancingRes(9) Ohm  i_specify=off  i_priority=None  i=0 A  i_nominal_specify=off  i_nominal_value=1  i_nominal_unit=A  v_specify=off  v_priority=None  v=0 V  v_nominal_specify=off  v_nominal_value=1  v_nominal_unit=V
  Resistor9 : fl_lib/Electrical/Electrical Elements/Resistor  R=PassiveBalancingRes(10) Ohm  i_specify=off  i_priority=None  i=0 A  i_nominal_specify=off  i_nominal_value=1  i_nominal_unit=A  v_specify=off  v_priority=None  v=0 V  v_nominal_specify=off  v_nominal_value=1  v_nominal_unit=V
  Selector : Selector  InputPortWidth=10  IndexOptions=Index vector (dialog)  Indices=1  OutputSizes=1
  Selector1 : Selector  InputPortWidth=10  IndexOptions=Index vector (dialog)  Indices=2  OutputSizes=1
  Selector2 : Selector  InputPortWidth=10  IndexOptions=Index vector (dialog)  Indices=3  OutputSizes=1
  Selector3 : Selector  InputPortWidth=10  IndexOptions=Index vector (dialog)  Indices=4  OutputSizes=1
  Selector4 : Selector  InputPortWidth=10  IndexOptions=Index vector (dialog)  Indices=5  OutputSizes=1
  Selector5 : Selector  InputPortWidth=10  IndexOptions=Index vector (dialog)  Indices=6  OutputSizes=1
  Selector6 : Selector  InputPortWidth=10  IndexOptions=Index vector (dialog)  Indices=7  OutputSizes=1
  Selector7 : Selector  InputPortWidth=10  IndexOptions=Index vector (dialog)  Indices=8  OutputSizes=1
  Selector8 : Selector  InputPortWidth=10  IndexOptions=Index vector (dialog)  Indices=9  OutputSizes=1
  Selector9 : Selector  InputPortWidth=10  IndexOptions=Index vector (dialog)  Indices=10  OutputSizes=1
  Simulink-PS Converter{,1..9} (10 alike) : nesl_utility/Simulink-PS Converter  PhysicalDomain=network_engine_domain  SubClassName=ps_input  LeftPortType=input  RightPortType=output  PseudoPeriodic=off  Frequencies=[]  NoiseDistribution=none  NoiseParameters=[]  Unit=1  AffineConversion=off  FilteringAndDerivatives=provide  SimscapeFilterOrder=1  InputFilterTimeConstant=0.001  UdotUserProvided=0
  Switch{,1..9} (10 alike) : fl_lib/Electrical/Electrical Elements/Switch  R_closed=0.01 Ohm  G_open=1e-8 1/Ohm  Threshold=0.5  i_specify=off  i_priority=None  i=0 A  i_nominal_specify=off  i_nominal_value=1  i_nominal_unit=A  v_specify=off  v_priority=None  v=0 V  v_nominal_specify=off  v_nominal_value=1  v_nominal_unit=V
  Vector Concatenate : Concatenate  NumInputs=10
  Voltage Sensor{,1..9} (10 alike) : fl_lib/Electrical/Electrical Sensors/Voltage Sensor
  V10 : PMIOPort  Side=Left
  V9 : PMIOPort  Port=2  Side=Left
  V8 : PMIOPort  Port=3  Side=Left
  V7 : PMIOPort  Port=4  Side=Left
  V6 : PMIOPort  Port=5  Side=Left
  V5 : PMIOPort  Port=6  Side=Left
  V4 : PMIOPort  Port=7  Side=Left
  V3 : PMIOPort  Port=8  Side=Left
  V2 : PMIOPort  Port=9  Side=Left
  V1 : PMIOPort  Port=10  Side=Left
  V0 : PMIOPort  Port=11  Side=Left
  VoltageVector : Outport
  wires:
  PS-Simulink Converter6/1 -> Vector Concatenate/7
  PS-Simulink Converter/1 -> Vector Concatenate/1
  PS-Simulink Converter7/1 -> Vector Concatenate/8
  PassiveControlBalCmd1/1 -> Bus Selector/1
  Selector7/1 -> Simulink-PS Converter7/1
  Selector/1 -> Simulink-PS Converter/1
  PS-Simulink Converter5/1 -> Vector Concatenate/6
  Selector2/1 -> Simulink-PS Converter2/1
  Selector1/1 -> Simulink-PS Converter1/1
  Selector6/1 -> Simulink-PS Converter6/1
  PS-Simulink Converter2/1 -> Vector Concatenate/3
  Vector Concatenate/1 -> VoltageVector/1
  PS-Simulink Converter9/1 -> Vector Concatenate/10
  Selector9/1 -> Simulink-PS Converter9/1
  Selector4/1 -> Simulink-PS Converter4/1
  PS-Simulink Converter1/1 -> Vector Concatenate/2
  PS-Simulink Converter4/1 -> Vector Concatenate/5
  PS-Simulink Converter3/1 -> Vector Concatenate/4
  Selector8/1 -> Simulink-PS Converter8/1
  PS-Simulink Converter8/1 -> Vector Concatenate/9
  Selector3/1 -> Simulink-PS Converter3/1
  Selector5/1 -> Simulink-PS Converter5/1
  Bus Selector/1 -> Data Type Conversion/1  "<balancingCmd>"
  Data Type Conversion/1 -> Selector2/1, Selector3/1, Selector4/1, Selector5/1, Selector6/1, Selector7/1, Selector8/1, Selector9/1, Selector1/1, Selector/1
  connections:
  Simulink-PS Converter9/RConn1 -- Switch9/RConn1
  Simulink-PS Converter8/RConn1 -- Switch8/RConn1
  Switch3/LConn1 -- Diode3/RConn1
  Simulink-PS Converter7/RConn1 -- Switch7/RConn1
  Switch6/LConn1 -- Diode6/RConn1
  Switch8/LConn1 -- Diode8/RConn1
  Simulink-PS Converter5/RConn1 -- Switch5/RConn1
  Voltage Sensor7/LConn1 -- V3/RConn1 -- Switch6/RConn2 -- Resistor7/LConn1 -- Voltage Sensor6/RConn2
  Resistor5/RConn1 -- Diode5/LConn1
  Voltage Sensor2/LConn1 -- V8/RConn1 -- Switch1/RConn2 -- Resistor2/LConn1 -- Voltage Sensor1/RConn2
  Voltage Sensor1/RConn1 -- PS-Simulink Converter1/LConn1
  Diode/LConn1 -- Resistor/RConn1
  Resistor9/RConn1 -- Diode9/LConn1
  Voltage Sensor6/LConn1 -- V4/RConn1 -- Switch5/RConn2 -- Resistor6/LConn1 -- Voltage Sensor5/RConn2
  Switch4/LConn1 -- Diode4/RConn1
  Simulink-PS Converter4/RConn1 -- Switch4/RConn1
  Voltage Sensor9/LConn1 -- V1/RConn1 -- Switch8/RConn2 -- Resistor9/LConn1 -- Voltage Sensor8/RConn2
  Voltage Sensor1/LConn1 -- V9/RConn1 -- Switch/RConn2 -- Resistor1/LConn1 -- Voltage Sensor/RConn2
  Voltage Sensor3/RConn1 -- PS-Simulink Converter3/LConn1
  Resistor2/RConn1 -- Diode2/LConn1
  Diode1/LConn1 -- Resistor1/RConn1
  Resistor8/RConn1 -- Diode8/LConn1
  Switch2/LConn1 -- Diode2/RConn1
  Switch7/LConn1 -- Diode7/RConn1
  Switch/LConn1 -- Diode/RConn1
  Voltage Sensor9/RConn1 -- PS-Simulink Converter9/LConn1
  Simulink-PS Converter3/RConn1 -- Switch3/RConn1
  PS-Simulink Converter/LConn1 -- Voltage Sensor/RConn1
  Voltage Sensor3/LConn1 -- V7/RConn1 -- Switch2/RConn2 -- Resistor3/LConn1 -- Voltage Sensor2/RConn2
  Voltage Sensor2/RConn1 -- PS-Simulink Converter2/LConn1
  Simulink-PS Converter6/RConn1 -- Switch6/RConn1
  Switch5/LConn1 -- Diode5/RConn1
  Voltage Sensor9/RConn2 -- V0/RConn1 -- Switch9/RConn2
  Simulink-PS Converter2/RConn1 -- Switch2/RConn1
  Simulink-PS Converter/RConn1 -- Switch/RConn1
  Voltage Sensor8/LConn1 -- V2/RConn1 -- Switch7/RConn2 -- Resistor8/LConn1 -- Voltage Sensor7/RConn2
  Voltage Sensor5/LConn1 -- V5/RConn1 -- Switch4/RConn2 -- Resistor5/LConn1 -- Voltage Sensor4/RConn2
  Switch9/LConn1 -- Diode9/RConn1
  Voltage Sensor4/LConn1 -- V6/RConn1 -- Switch3/RConn2 -- Resistor4/LConn1 -- Voltage Sensor3/RConn2
  Resistor3/RConn1 -- Diode3/LConn1
  Voltage Sensor/LConn1 -- V10/RConn1 -- Resistor/LConn1
  Resistor6/RConn1 -- Diode6/LConn1
  Switch1/LConn1 -- Diode1/RConn1
  Voltage Sensor6/RConn1 -- PS-Simulink Converter6/LConn1
  Voltage Sensor7/RConn1 -- PS-Simulink Converter7/LConn1
  Voltage Sensor8/RConn1 -- PS-Simulink Converter8/LConn1
  Simulink-PS Converter1/RConn1 -- Switch1/RConn1
  Voltage Sensor5/RConn1 -- PS-Simulink Converter5/LConn1
  Voltage Sensor4/RConn1 -- PS-Simulink Converter4/LConn1
  Resistor7/RConn1 -- Diode7/LConn1
  Resistor4/RConn1 -- Diode4/LConn1
